simulink model "gate_V36_2016a_SIM"  (R2016a) (continued)
== system gate_V36_2016a_SIM/Gate/HardwareInputWrapper/HIL/BluetoothWrapper ==
  S-Function Builder : S-Function  InitFcn=try, set_param(gcb,'FunctionName','BluetoothLib'), end  FunctionName=BluetoothLib  SFunctionModules=BluetoothLib_wrapper  SFunctionDeploymentMode=off  EnableBusSupport=off  mask(S-Function Builder){}
      mask help: Simulink:masks:SFunctionBuilder_MD
      mask init: same as gate_V36_2016a_SIM/Gate/HardwareInputWrapper/HIL/S-Function Builder1
      settings: CopyFcn=set_param(gcbh,'LinkStatus','none')  PreSaveFcn=try, set_param(gcb,'FunctionName','BluetoothLib'), end  OpenFcn=sfunctionwizard(gcbh)
      mask Display: plot(val(:,1),val(:,2)),disp(sys), port_label('output',1,'BtCharRecieved')
  S-Function Builder1 : S-Function  Commented=on  InitFcn=try, set_param(gcb,'FunctionName','printingBlock'), end  FunctionName=printingBlock  SFunctionModules=printingBlock_wrapper  SFunctionDeploymentMode=off  EnableBusSupport=off  mask(S-Function Builder){}
      mask help: Simulink:masks:SFunctionBuilder_MD
      mask init: same as gate_V36_2016a_SIM/Gate/HardwareInputWrapper/HIL/S-Function Builder1
      settings: CopyFcn=set_param(gcbh,'LinkStatus','none')  PreSaveFcn=try, set_param(gcb,'FunctionName','printingBlock'), end  OpenFcn=sfunctionwizard(gcbh)
      mask Display: plot(val(:,1),val(:,2)),disp(sys), port_label('input',1,'u0'), port_label('output',1,'y0')
  Unit Delay : UnitDelay  Commented=on  SampleTime=-1
  bluetoothCharPressed : Outport
  wires:
  S-Function Builder/1 -> S-Function Builder1/1, Unit Delay/1, bluetoothCharPressed/1
  Unit Delay/1 -> ?

== system gate_V36_2016a_SIM/Gate/HardwareInputWrapper/HIL/ButtonsPressed ==
  S-Function Builder1 : S-Function  InitFcn=try, set_param(gcb,'FunctionName','Button_Lib'), end  FunctionName=Button_Lib  SFunctionModules=Button_Lib_wrapper  SFunctionDeploymentMode=off  EnableBusSupport=off  mask(S-Function Builder){}
      mask help: Simulink:masks:SFunctionBuilder_MD
      mask init: same as gate_V36_2016a_SIM/Gate/HardwareInputWrapper/HIL/S-Function Builder1
      settings: CopyFcn=set_param(gcbh,'LinkStatus','none')  PreSaveFcn=try, set_param(gcb,'FunctionName','Button_Lib'), end  OpenFcn=sfunctionwizard(gcbh)
      mask Display: plot(val(:,1),val(:,2)),disp(sys), port_label('output',1,'RFInput'), port_label('output',2,'passSw')
  RFPressed : Outport
  SWPressed : Outport  Port=2
  wires:
  S-Function Builder1/1 -> RFPressed/1
  S-Function Builder1/2 -> SWPressed/1

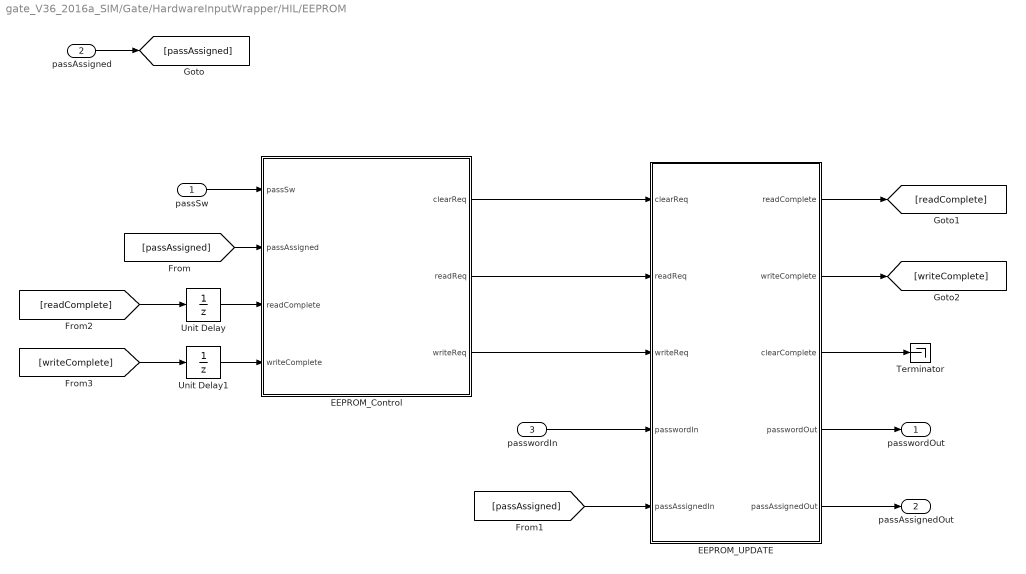
== system gate_V36_2016a_SIM/Gate/HardwareInputWrapper/HIL/EEPROM ==
  passSw : Inport
  passAssigned : Inport  Port=2
  passwordIn : Inport  Port=3
  EEPROM_Control : Stateflow chart  TreatAsAtomicUnit=on
  EEPROM_UPDATE : Subsystem  TreatAsAtomicUnit=on
  Terminator : Terminator
  Unit Delay : UnitDelay  SampleTime=-1
  Unit Delay1 : UnitDelay  SampleTime=-1
  passwordOut : Outport
  passAssignedOut : Outport  Port=2
  wires:
  EEPROM_UPDATE/3(clearComplete) -> Terminator/1
  EEPROM_UPDATE/5(passAssignedOut) -> passAssignedOut/1
  EEPROM_UPDATE/4(passwordOut) -> passwordOut/1
  EEPROM_UPDATE/2(writeComplete) -> [writeComplete]
  [writeComplete] -> Unit Delay1/1
  [readComplete] -> Unit Delay/1
  EEPROM_UPDATE/1(readComplete) -> [readComplete]
  passAssigned/1 -> [passAssigned]
  [passAssigned] -> EEPROM_UPDATE/5(passAssignedIn)
  [passAssigned] -> EEPROM_Control/2(passAssigned)
  passwordIn/1 -> EEPROM_UPDATE/4(passwordIn)
  EEPROM_Control/3(writeReq) -> EEPROM_UPDATE/3(writeReq)
  EEPROM_Control/2(readReq) -> EEPROM_UPDATE/2(readReq)
  EEPROM_Control/1(clearReq) -> EEPROM_UPDATE/1(clearReq)
  Unit Delay1/1 -> EEPROM_Control/4(writeComplete)
  Unit Delay/1 -> EEPROM_Control/3(readComplete)
  passSw/1 -> EEPROM_Control/1(passSw)

== system gate_V36_2016a_SIM/Gate/HardwareInputWrapper/HIL/KeypadWrapper ==
  KeypadLib : S-Function  InitFcn=try, set_param(gcb,'FunctionName','keypadLib'), end  FunctionName=keypadLib  SFunctionModules=keypadLib_wrapper  SFunctionDeploymentMode=off  EnableBusSupport=off  mask(S-Function Builder){}
      mask help: Simulink:masks:SFunctionBuilder_MD
      mask init: same as gate_V36_2016a_SIM/Gate/HardwareInputWrapper/HIL/S-Function Builder1
      settings: CopyFcn=set_param(gcbh,'LinkStatus','none')  PreSaveFcn=try, set_param(gcb,'FunctionName','keypadLib'), end  OpenFcn=sfunctionwizard(gcbh)
      mask Display: plot(val(:,1),val(:,2)),disp(sys), port_label('output',1,'charPressed')
  charPressed : Outport
  wires:
  KeypadLib/1 -> charPressed/1

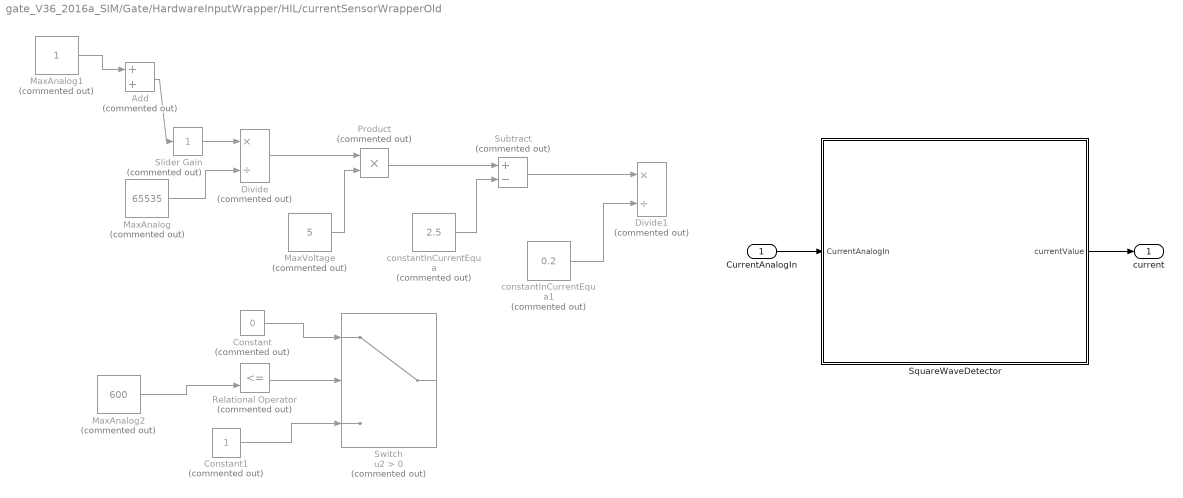
== system gate_V36_2016a_SIM/Gate/HardwareInputWrapper/HIL/currentSensorWrapperOld ==
  CurrentAnalogIn : Inport
  Add : Sum  Commented=on
  Constant : Constant  Commented=on  Value=0
  Constant1 : Constant  Commented=on
  Divide : Product  Commented=on  Inputs=*/
  Divide1 : Product  Commented=on  Inputs=*/
  MaxAnalog : Constant  Commented=on  Value=65535
  MaxAnalog1 : Constant  Commented=on
  MaxAnalog2 : Constant  Commented=on  Value=600
  MaxVoltage : Constant  Commented=on  Value=5
  Product : Product  Commented=on
  Relational Operator : RelationalOperator  Commented=on  Operator=<=  OutDataTypeStr=boolean
  Slider Gain : simulink/Math Operations/Slider Gain  Commented=on  low=0  gain=1  high=255
  SquareWaveDetector : Stateflow chart  TreatAsAtomicUnit=on
  Subtract : Sum  Commented=on  Inputs=+-
  Switch : Switch  Commented=on  Criteria=u2 > Threshold
  constantInCurrentEqua : Constant  Commented=on  Value=2.5
  constantInCurrentEqua1 : Constant  Commented=on  Value=0.2
  current : Outport
  wires:
  MaxAnalog/1 -> Divide/2
  Divide/1 -> Product/1
  MaxVoltage/1 -> Product/2
  Product/1 -> Subtract/1
  constantInCurrentEqua/1 -> Subtract/2
  Subtract/1 -> Divide1/1
  constantInCurrentEqua1/1 -> Divide1/2
  Slider Gain/1 -> Divide/1
  Add/1 -> Slider Gain/1
  MaxAnalog1/1 -> Add/1
  MaxAnalog2/1 -> Relational Operator/2
  Relational Operator/1 -> Switch/2
  Constant1/1 -> Switch/3
  Constant/1 -> Switch/1
  CurrentAnalogIn/1 -> SquareWaveDetector/1(CurrentAnalogIn)
  SquareWaveDetector/1(currentValue) -> current/1

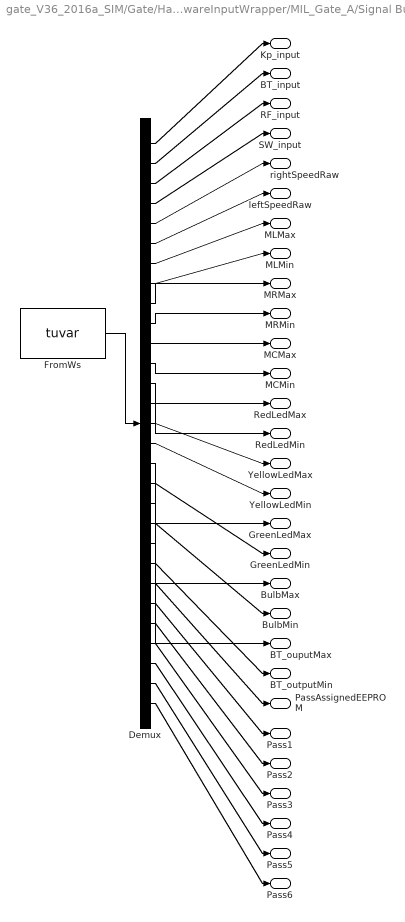
== system gate_V36_2016a_SIM/Gate/HardwareInputWrapper/MIL_Gate_A/Signal Builder ==
  Demux : Demux  Tag=STV Demux  Outputs=29
  FromWs : FromWorkspace  Tag=STV FromWs  VariableName=tuvar  SampleTime=0
  Kp_input : Outport  Tag=STV Outport
  BT_input : Outport  Tag=STV Outport  Port=2
  RF_input : Outport  Tag=STV Outport  Port=3
  SW_input : Outport  Tag=STV Outport  Port=4
  rightSpeedRaw : Outport  Tag=STV Outport  Port=5
  leftSpeedRaw : Outport  Tag=STV Outport  Port=6
  MLMax : Outport  Tag=STV Outport  Port=7
  MLMin : Outport  Tag=STV Outport  Port=8
  MRMax : Outport  Tag=STV Outport  Port=9
  MRMin : Outport  Tag=STV Outport  Port=10
  MCMax : Outport  Tag=STV Outport  Port=11
  MCMin : Outport  Tag=STV Outport  Port=12
  RedLedMax : Outport  Tag=STV Outport  Port=13
  RedLedMin : Outport  Tag=STV Outport  Port=14
  YellowLedMax : Outport  Tag=STV Outport  Port=15
  YellowLedMin : Outport  Tag=STV Outport  Port=16
  GreenLedMax : Outport  Tag=STV Outport  Port=17
  GreenLedMin : Outport  Tag=STV Outport  Port=18
  BulbMax : Outport  Tag=STV Outport  Port=19
  BulbMin : Outport  Tag=STV Outport  Port=20
  BT_ouputMax : Outport  Tag=STV Outport  Port=21
  BT_outputMin : Outport  Tag=STV Outport  Port=22
  PassAssignedEEPROM : Outport  Tag=STV Outport  Port=23
  Pass1 : Outport  Tag=STV Outport  Port=24
  Pass2 : Outport  Tag=STV Outport  Port=25
  Pass3 : Outport  Tag=STV Outport  Port=26
  Pass4 : Outport  Tag=STV Outport  Port=27
  Pass5 : Outport  Tag=STV Outport  Port=28
  Pass6 : Outport  Tag=STV Outport  Port=29
  wires:
  FromWs/1 -> Demux/1
  Demux/1 -> Kp_input/1
  Demux/2 -> BT_input/1
  Demux/3 -> RF_input/1
  Demux/4 -> SW_input/1
  Demux/5 -> rightSpeedRaw/1
  Demux/6 -> leftSpeedRaw/1
  Demux/7 -> MLMax/1
  Demux/8 -> MLMin/1
  Demux/9 -> MRMax/1
  Demux/10 -> MRMin/1
  Demux/11 -> MCMax/1
  Demux/12 -> MCMin/1
  Demux/13 -> RedLedMax/1
  Demux/14 -> RedLedMin/1
  Demux/15 -> YellowLedMax/1
  Demux/16 -> YellowLedMin/1
  Demux/17 -> GreenLedMax/1
  Demux/18 -> GreenLedMin/1
  Demux/19 -> BulbMax/1
  Demux/20 -> BulbMin/1
  Demux/21 -> BT_ouputMax/1
  Demux/22 -> BT_outputMin/1
  Demux/23 -> PassAssignedEEPROM/1
  Demux/24 -> Pass1/1
  Demux/25 -> Pass2/1
  Demux/26 -> Pass3/1
  Demux/27 -> Pass4/1
  Demux/28 -> Pass5/1
  Demux/29 -> Pass6/1

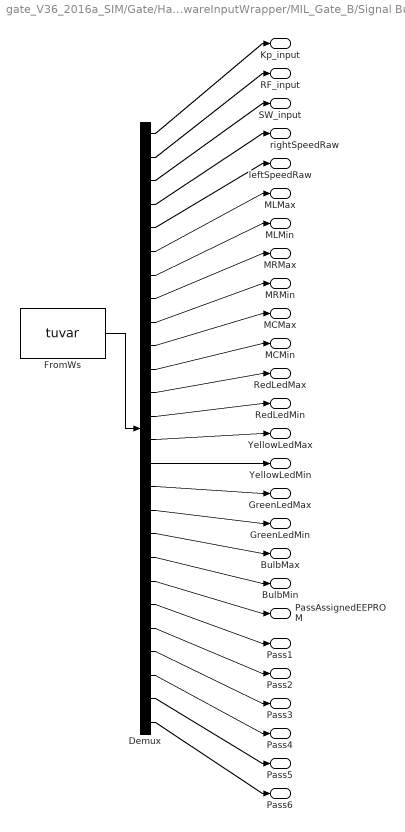
== system gate_V36_2016a_SIM/Gate/HardwareInputWrapper/MIL_Gate_B/Signal Builder ==
  Demux : Demux  Tag=STV Demux  Outputs=26
  FromWs : FromWorkspace  Tag=STV FromWs  VariableName=tuvar  SampleTime=0  OutputAfterFinalValue=Holding final value
  Kp_input : Outport  Tag=STV Outport
  RF_input : Outport  Tag=STV Outport  Port=2
  SW_input : Outport  Tag=STV Outport  Port=3
  rightSpeedRaw : Outport  Tag=STV Outport  Port=4
  leftSpeedRaw : Outport  Tag=STV Outport  Port=5
  MLMax : Outport  Tag=STV Outport  Port=6
  MLMin : Outport  Tag=STV Outport  Port=7
  MRMax : Outport  Tag=STV Outport  Port=8
  MRMin : Outport  Tag=STV Outport  Port=9
  MCMax : Outport  Tag=STV Outport  Port=10
  MCMin : Outport  Tag=STV Outport  Port=11
  RedLedMax : Outport  Tag=STV Outport  Port=12
  RedLedMin : Outport  Tag=STV Outport  Port=13
  YellowLedMax : Outport  Tag=STV Outport  Port=14
  YellowLedMin : Outport  Tag=STV Outport  Port=15
  GreenLedMax : Outport  Tag=STV Outport  Port=16
  GreenLedMin : Outport  Tag=STV Outport  Port=17
  BulbMax : Outport  Tag=STV Outport  Port=18
  BulbMin : Outport  Tag=STV Outport  Port=19
  PassAssignedEEPROM : Outport  Tag=STV Outport  Port=20
  Pass1 : Outport  Tag=STV Outport  Port=21
  Pass2 : Outport  Tag=STV Outport  Port=22
  Pass3 : Outport  Tag=STV Outport  Port=23
  Pass4 : Outport  Tag=STV Outport  Port=24
  Pass5 : Outport  Tag=STV Outport  Port=25
  Pass6 : Outport  Tag=STV Outport  Port=26
  wires:
  FromWs/1 -> Demux/1
  Demux/1 -> Kp_input/1
  Demux/2 -> RF_input/1
  Demux/3 -> SW_input/1
  Demux/4 -> rightSpeedRaw/1
  Demux/5 -> leftSpeedRaw/1
  Demux/6 -> MLMax/1
  Demux/7 -> MLMin/1
  Demux/8 -> MRMax/1
  Demux/9 -> MRMin/1
  Demux/10 -> MCMax/1
  Demux/11 -> MCMin/1
  Demux/12 -> RedLedMax/1
  Demux/13 -> RedLedMin/1
  Demux/14 -> YellowLedMax/1
  Demux/15 -> YellowLedMin/1
  Demux/16 -> GreenLedMax/1
  Demux/17 -> GreenLedMin/1
  Demux/18 -> BulbMax/1
  Demux/19 -> BulbMin/1
  Demux/20 -> PassAssignedEEPROM/1
  Demux/21 -> Pass1/1
  Demux/22 -> Pass2/1
  Demux/23 -> Pass3/1
  Demux/24 -> Pass4/1
  Demux/25 -> Pass5/1
  Demux/26 -> Pass6/1

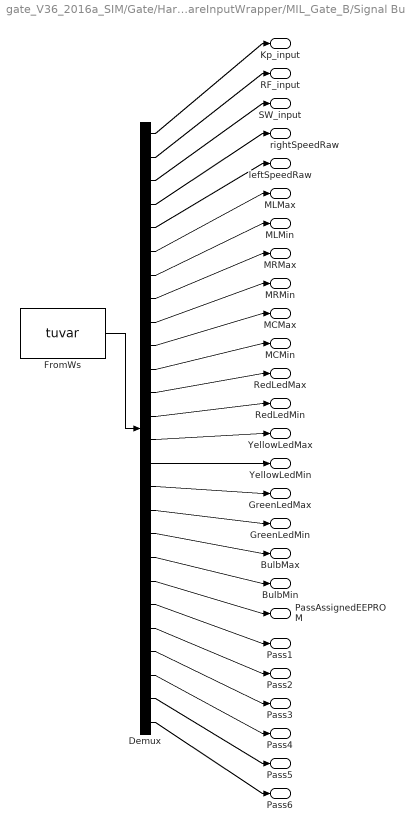
== system gate_V36_2016a_SIM/Gate/HardwareInputWrapper/MIL_Gate_B/Signal Builder1 ==
  Demux : Demux  Tag=STV Demux  Outputs=26
  FromWs : FromWorkspace  Tag=STV FromWs  VariableName=tuvar  SampleTime=0  OutputAfterFinalValue=Holding final value
  Kp_input : Outport  Tag=STV Outport
  RF_input : Outport  Tag=STV Outport  Port=2
  SW_input : Outport  Tag=STV Outport  Port=3
  rightSpeedRaw : Outport  Tag=STV Outport  Port=4
  leftSpeedRaw : Outport  Tag=STV Outport  Port=5
  MLMax : Outport  Tag=STV Outport  Port=6
  MLMin : Outport  Tag=STV Outport  Port=7
  MRMax : Outport  Tag=STV Outport  Port=8
  MRMin : Outport  Tag=STV Outport  Port=9
  MCMax : Outport  Tag=STV Outport  Port=10
  MCMin : Outport  Tag=STV Outport  Port=11
  RedLedMax : Outport  Tag=STV Outport  Port=12
  RedLedMin : Outport  Tag=STV Outport  Port=13
  YellowLedMax : Outport  Tag=STV Outport  Port=14
  YellowLedMin : Outport  Tag=STV Outport  Port=15
  GreenLedMax : Outport  Tag=STV Outport  Port=16
  GreenLedMin : Outport  Tag=STV Outport  Port=17
  BulbMax : Outport  Tag=STV Outport  Port=18
  BulbMin : Outport  Tag=STV Outport  Port=19
  PassAssignedEEPROM : Outport  Tag=STV Outport  Port=20
  Pass1 : Outport  Tag=STV Outport  Port=21
  Pass2 : Outport  Tag=STV Outport  Port=22
  Pass3 : Outport  Tag=STV Outport  Port=23
  Pass4 : Outport  Tag=STV Outport  Port=24
  Pass5 : Outport  Tag=STV Outport  Port=25
  Pass6 : Outport  Tag=STV Outport  Port=26
  wires:
  Demux/26 -> Pass6/1
  Demux/25 -> Pass5/1
  Demux/24 -> Pass4/1
  Demux/23 -> Pass3/1
  Demux/22 -> Pass2/1
  Demux/21 -> Pass1/1
  Demux/20 -> PassAssignedEEPROM/1
  Demux/19 -> BulbMin/1
  Demux/18 -> BulbMax/1
  Demux/17 -> GreenLedMin/1
  Demux/16 -> GreenLedMax/1
  Demux/15 -> YellowLedMin/1
  Demux/14 -> YellowLedMax/1
  Demux/13 -> RedLedMin/1
  Demux/12 -> RedLedMax/1
  Demux/11 -> MCMin/1
  Demux/10 -> MCMax/1
  Demux/9 -> MRMin/1
  Demux/8 -> MRMax/1
  Demux/7 -> MLMin/1
  Demux/6 -> MLMax/1
  Demux/5 -> leftSpeedRaw/1
  Demux/4 -> rightSpeedRaw/1
  Demux/3 -> SW_input/1
  Demux/2 -> RF_input/1
  Demux/1 -> Kp_input/1
  FromWs/1 -> Demux/1

== system gate_V36_2016a_SIM/Gate/HardwareInputWrapper/MIL_Gate_C/Signal Builder ==
  Demux : Demux  Tag=STV Demux  Outputs=12
  FromWs : FromWorkspace  Tag=STV FromWs  VariableName=tuvar  SampleTime=0
  RF_input : Outport  Tag=STV Outport
  SW_input : Outport  Tag=STV Outport  Port=2
  rightSpeedRaw : Outport  Tag=STV Outport  Port=3
  leftSpeedRaw : Outport  Tag=STV Outport  Port=4
  MLMax : Outport  Tag=STV Outport  Port=5
  MLMin : Outport  Tag=STV Outport  Port=6
  MRMax : Outport  Tag=STV Outport  Port=7
  MRMin : Outport  Tag=STV Outport  Port=8
  MCMax : Outport  Tag=STV Outport  Port=9
  MCMin : Outport  Tag=STV Outport  Port=10
  BulbMax : Outport  Tag=STV Outport  Port=11
  BulbMin : Outport  Tag=STV Outport  Port=12
  wires:
  FromWs/1 -> Demux/1
  Demux/1 -> RF_input/1
  Demux/2 -> SW_input/1
  Demux/3 -> rightSpeedRaw/1
  Demux/4 -> leftSpeedRaw/1
  Demux/5 -> MLMax/1
  Demux/6 -> MLMin/1
  Demux/7 -> MRMax/1
  Demux/8 -> MRMin/1
  Demux/9 -> MCMax/1
  Demux/10 -> MCMin/1
  Demux/11 -> BulbMax/1
  Demux/12 -> BulbMin/1

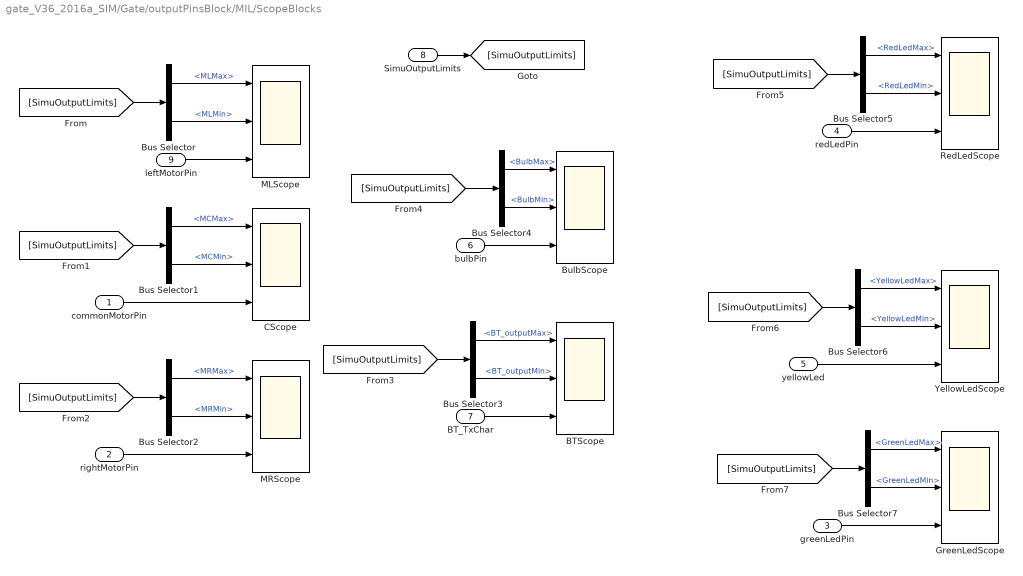
== system gate_V36_2016a_SIM/Gate/outputPinsBlock/MIL/ScopeBlocks ==
  commonMotorPin : Inport
  rightMotorPin : Inport  Port=2
  greenLedPin : Inport  Port=3
  redLedPin : Inport  Port=4
  yellowLed : Inport  Port=5
  bulbPin : Inport  Port=6
  BT_TxChar : Inport  Port=7
  SimuOutputLimits : Inport  Port=8
  leftMotorPin : Inport  Port=9
  BTScope : Scope  NumInputPorts=3
  BulbScope : Scope  NumInputPorts=3
  Bus Selector : BusSelector  OutputSignals=MLMax,MLMin
  Bus Selector1 : BusSelector  OutputSignals=MCMax,MCMin
  Bus Selector2 : BusSelector  OutputSignals=MRMax,MRMin
  Bus Selector3 : BusSelector  OutputSignals=BT_outputMax,BT_outputMin
  Bus Selector4 : BusSelector  OutputSignals=BulbMax,BulbMin
  Bus Selector5 : BusSelector  OutputSignals=RedLedMax,RedLedMin
  Bus Selector6 : BusSelector  OutputSignals=YellowLedMax,YellowLedMin
  Bus Selector7 : BusSelector  OutputSignals=GreenLedMax,GreenLedMin
  CScope : Scope  NumInputPorts=3
  GreenLedScope : Scope  NumInputPorts=3
  MLScope : Scope  NumInputPorts=3
  MRScope : Scope  NumInputPorts=3
  RedLedScope : Scope  NumInputPorts=3
  YellowLedScope : Scope  NumInputPorts=3
  wires:
  rightMotorPin/1 -> MRScope/3
  commonMotorPin/1 -> CScope/3
  bulbPin/1 -> BulbScope/3
  greenLedPin/1 -> GreenLedScope/3
  yellowLed/1 -> YellowLedScope/3
  redLedPin/1 -> RedLedScope/3
  BT_TxChar/1 -> BTScope/3
  SimuOutputLimits/1 -> [SimuOutputLimits]
  [SimuOutputLimits] -> Bus Selector/1
  leftMotorPin/1 -> MLScope/3
  Bus Selector/1 -> MLScope/1  "<MLMax>"
  Bus Selector/2 -> MLScope/2  "<MLMin>"
  [SimuOutputLimits] -> Bus Selector1/1
  Bus Selector1/1 -> CScope/1  "<MCMax>"
  Bus Selector1/2 -> CScope/2  "<MCMin>"
  [SimuOutputLimits] -> Bus Selector2/1
  Bus Selector2/1 -> MRScope/1  "<MRMax>"
  Bus Selector2/2 -> MRScope/2  "<MRMin>"
  [SimuOutputLimits] -> Bus Selector3/1
  Bus Selector3/1 -> BTScope/1  "<BT_outputMax>"
  Bus Selector3/2 -> BTScope/2  "<BT_outputMin>"
  [SimuOutputLimits] -> Bus Selector4/1
  Bus Selector4/1 -> BulbScope/1  "<BulbMax>"
  Bus Selector4/2 -> BulbScope/2  "<BulbMin>"
  [SimuOutputLimits] -> Bus Selector5/1
  Bus Selector5/1 -> RedLedScope/1  "<RedLedMax>"
  Bus Selector5/2 -> RedLedScope/2  "<RedLedMin>"
  [SimuOutputLimits] -> Bus Selector6/1
  Bus Selector6/1 -> YellowLedScope/1  "<YellowLedMax>"
  Bus Selector6/2 -> YellowLedScope/2  "<YellowLedMin>"
  [SimuOutputLimits] -> Bus Selector7/1
  Bus Selector7/1 -> GreenLedScope/1  "<GreenLedMax>"
  Bus Selector7/2 -> GreenLedScope/2  "<GreenLedMin>"

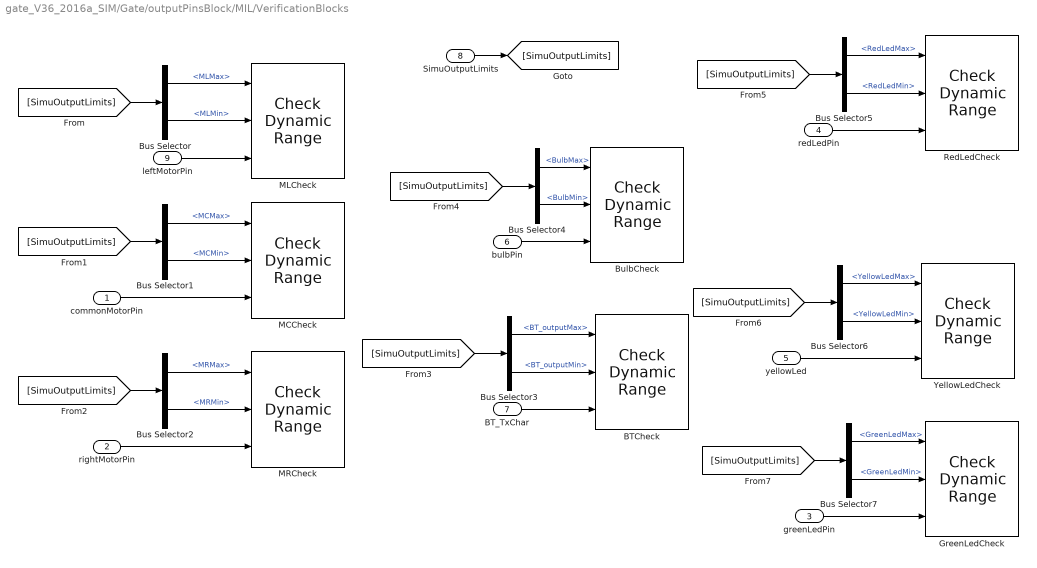
== system gate_V36_2016a_SIM/Gate/outputPinsBlock/MIL/VerificationBlocks  ==
  commonMotorPin : Inport
  rightMotorPin : Inport  Port=2
  greenLedPin : Inport  Port=3
  redLedPin : Inport  Port=4
  yellowLed : Inport  Port=5
  bulbPin : Inport  Port=6
  BT_TxChar : Inport  Port=7
  SimuOutputLimits : Inport  Port=8
  leftMotorPin : Inport  Port=9
  BTCheck : simulink/Model Verification/Check  Dynamic Range  Description=min < sig < max  enabled=on  stopWhenAssertionFail=on  export=off  icon=graphic
  BulbCheck : simulink/Model Verification/Check  Dynamic Range  Description=min < sig < max  enabled=on  stopWhenAssertionFail=on  export=off  icon=graphic
  Bus Selector : BusSelector  OutputSignals=MLMax,MLMin
  Bus Selector1 : BusSelector  OutputSignals=MCMax,MCMin
  Bus Selector2 : BusSelector  OutputSignals=MRMax,MRMin
  Bus Selector3 : BusSelector  OutputSignals=BT_outputMax,BT_outputMin
  Bus Selector4 : BusSelector  OutputSignals=BulbMax,BulbMin
  Bus Selector5 : BusSelector  OutputSignals=RedLedMax,RedLedMin
  Bus Selector6 : BusSelector  OutputSignals=YellowLedMax,YellowLedMin
  Bus Selector7 : BusSelector  OutputSignals=GreenLedMax,GreenLedMin
  GreenLedCheck : simulink/Model Verification/Check  Dynamic Range  Description=min < sig < max  enabled=on  stopWhenAssertionFail=on  export=off  icon=graphic
  MCCheck : simulink/Model Verification/Check  Dynamic Range  Description=min < sig < max  enabled=on  stopWhenAssertionFail=on  export=off  icon=graphic
  MLCheck : simulink/Model Verification/Check  Dynamic Range  Description=min < sig < max  enabled=on  stopWhenAssertionFail=on  export=off  icon=graphic
  MRCheck : simulink/Model Verification/Check  Dynamic Range  Description=min < sig < max  enabled=on  stopWhenAssertionFail=on  export=off  icon=graphic
  RedLedCheck : simulink/Model Verification/Check  Dynamic Range  Description=min < sig < max  enabled=on  stopWhenAssertionFail=on  export=off  icon=graphic
  YellowLedCheck : simulink/Model Verification/Check  Dynamic Range  Description=min < sig < max  enabled=on  stopWhenAssertionFail=on  export=off  icon=graphic
  wires:
  rightMotorPin/1 -> MRCheck/3
  commonMotorPin/1 -> MCCheck/3
  bulbPin/1 -> BulbCheck/3
  greenLedPin/1 -> GreenLedCheck/3
  yellowLed/1 -> YellowLedCheck/3
  redLedPin/1 -> RedLedCheck/3
  BT_TxChar/1 -> BTCheck/3
  SimuOutputLimits/1 -> [SimuOutputLimits]
  [SimuOutputLimits] -> Bus Selector/1
  leftMotorPin/1 -> MLCheck/3
  Bus Selector/1 -> MLCheck/1  "<MLMax>"
  Bus Selector/2 -> MLCheck/2  "<MLMin>"
  [SimuOutputLimits] -> Bus Selector1/1
  Bus Selector1/1 -> MCCheck/1  "<MCMax>"
  Bus Selector1/2 -> MCCheck/2  "<MCMin>"
  [SimuOutputLimits] -> Bus Selector2/1
  Bus Selector2/1 -> MRCheck/1  "<MRMax>"
  Bus Selector2/2 -> MRCheck/2  "<MRMin>"
  [SimuOutputLimits] -> Bus Selector3/1
  Bus Selector3/1 -> BTCheck/1  "<BT_outputMax>"
  Bus Selector3/2 -> BTCheck/2  "<BT_outputMin>"
  [SimuOutputLimits] -> Bus Selector4/1
  Bus Selector4/1 -> BulbCheck/1  "<BulbMax>"
  Bus Selector4/2 -> BulbCheck/2  "<BulbMin>"
  [SimuOutputLimits] -> Bus Selector5/1
  Bus Selector5/1 -> RedLedCheck/1  "<RedLedMax>"
  Bus Selector5/2 -> RedLedCheck/2  "<RedLedMin>"
  [SimuOutputLimits] -> Bus Selector6/1
  Bus Selector6/1 -> YellowLedCheck/1  "<YellowLedMax>"
  Bus Selector6/2 -> YellowLedCheck/2  "<YellowLedMin>"
  [SimuOutputLimits] -> Bus Selector7/1
  Bus Selector7/1 -> GreenLedCheck/1  "<GreenLedMax>"
  Bus Selector7/2 -> GreenLedCheck/2  "<GreenLedMin>"

== system gate_V36_2016a_SIM/Gate/HardwareInputWrapper/HIL/EEPROM/EEPROM_UPDATE ==
  clearReq : Inport
  readReq : Inport  Port=2
  writeReq : Inport  Port=3
  passwordIn : Inport  Port=4
  passAssignedIn : Inport  Port=5
  S-Function Builder : S-Function  InitFcn=try, set_param(gcb,'FunctionName','myEEPROM_Handler'), end  FunctionName=myEEPROM_Handler  SFunctionModules=myEEPROM_Handler_wrapper  SFunctionDeploymentMode=off  EnableBusSupport=off  mask(S-Function Builder){}
      mask help: Simulink:masks:SFunctionBuilder_MD
      mask init: same as gate_V36_2016a_SIM/Gate/HardwareInputWrapper/HIL/S-Function Builder1
      settings: CopyFcn=set_param(gcbh,'LinkStatus','none')  PreSaveFcn=try, set_param(gcb,'FunctionName','myEEPROM_Handler'), end  OpenFcn=sfunctionwizard(gcbh)
      mask Display: plot(val(:,1),val(:,2)),disp(sys), port_label('input',1,'passwordIn'), port_label('input',2,'readReq'), port_label('input',3,'oldReadReq'), port_label('input',4,'clearReq'), port_label('input',5,'oldClearReq'), port_label('input',6,'writeReq'), port_label('input',7,'oldWriteReq'), port_label('input',8,'passAssignedIn'), port_label('output',1,'passwordOut'), port_label('output',2,'readComplete'), port_label('output',3,'writeComplete'), port_label('output',4,'clearComplete'), port_label('output',5,'passAssignedOut')
  Unit Delay : UnitDelay  SampleTime=-1
  Unit Delay1 : UnitDelay  SampleTime=-1
  Unit Delay2 : UnitDelay  SampleTime=-1
  readComplete : Outport
  writeComplete : Outport  Port=2
  clearComplete : Outport  Port=3
  passwordOut : Outport  Port=4
  passAssignedOut : Outport  Port=5
  wires:
  S-Function Builder/5 -> passAssignedOut/1
  S-Function Builder/1 -> passwordOut/1
  Unit Delay1/1 -> S-Function Builder/5
  Unit Delay2/1 -> S-Function Builder/7
  writeReq/1 -> S-Function Builder/6, Unit Delay2/1
  passAssignedIn/1 -> S-Function Builder/8
  clearReq/1 -> S-Function Builder/4, Unit Delay1/1
  Unit Delay/1 -> S-Function Builder/3
  readReq/1 -> S-Function Builder/2, Unit Delay/1
  passwordIn/1 -> S-Function Builder/1
  S-Function Builder/4 -> clearComplete/1
  S-Function Builder/2 -> readComplete/1
  S-Function Builder/3 -> writeComplete/1

== stateflow chart gate_V36_2016a_SIM/Gate/HardwareInputWrapper/HIL/EEPROM/EEPROM_Control ==
  state Idle
  state sendData
    entry:
    readReq = true;
  state Read
    entry:
    readReq = false;
  state write
    entry:
    writeReq = true;
  state clear
    entry:
    clearReq = true;
  Read -> sendData
  sendData -> Idle : [readComplete]/readReq = false;
  clear -> Idle [order 2] : [(hasChanged(passSw))...
      |  && (passSw == false)...
      | && (passAssigned == false)]
      | /clearReq = false;
  Idle -> clear : [(hasChanged(passSw))...
      |  && (passSw == true)]
  (default) -> Read
  write -> Read : [writeComplete]/writeReq = false;
  clear -> write [order 1] : [(hasChanged(passSw))...
      |  && (passSw == false)...
      | && (passAssigned == true)]
      | /clearReq = false;
  data: passSw input; passAssigned input; readComplete input; writeComplete input; clearReq output boolean =false; readReq output boolean =false; writeReq output boolean =false

== stateflow chart gate_V36_2016a_SIM/Gate/HardwareInputWrapper/HIL/currentSensorWrapperOld/SquareWaveDetector ==
  state Running
    entry:
    counterHigh = 0;
    counterLow= 0;
    currentValue = 1;
    state High
    state Low
    (default) -> High
    High -> High [order 2] : [CurrentAnalogIn > 1000]
        | /counterHigh++;
    High -> Low [order 1] : [CurrentAnalogIn < 1000]
    Low -> High [order 1] : [CurrentAnalogIn > 1000]
    Low -> Low [order 2] : [CurrentAnalogIn < 1000]
        | /counterLow++
  state Stop
    entry:
    currentValue = 0;
  (default) -> Stop
  Stop -> Running : [CurrentAnalogIn > 1000]
  Running -> Stop : [(counterHigh >= 200)...
      |  || (counterLow >= 200)]
  data: CurrentAnalogIn input; currentValue output uint8; counterHigh local uint16; counterLow local uint16
